simulink model "mbf_3beams"  (R2013a)
settings: Solver=VariableStepDiscrete  StartTime=0.0  StopTime=1000  RelTol=1e-3
undefined symbols (not defined in the file): pfb_coeff_gen_calc
libraries: xbsIndex_r4 x21411, casper_library_misc x306, casper_library_delays x93, casper_library_multipliers x64, casper_library_pfbs x16, simulink x16, xbsReplacements_r4 x8, xps_library x1
(part 3 of 254)

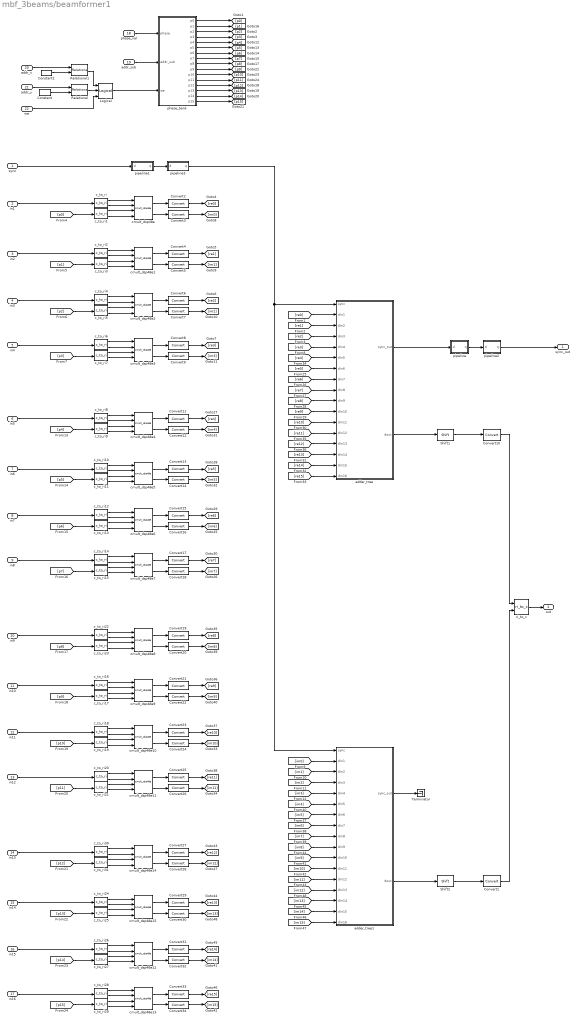
== system mbf_3beams/beamformer1 ==
  sync : Inport
  in1 : Inport  Port=2
  in2 : Inport  Port=3
  in3 : Inport  Port=4
  in4 : Inport  Port=5
  in5 : Inport  Port=6
  in6 : Inport  Port=7
  in7 : Inport  Port=8
  in8 : Inport  Port=9
  in9 : Inport  Port=10
  in10 : Inport  Port=11
  in11 : Inport  Port=12
  in12 : Inport  Port=13
  in13 : Inport  Port=14
  in14 : Inport  Port=15
  in15 : Inport  Port=16
  in16 : Inport  Port=17
  phase_val : Inport  Port=18
  addr_sub : Inport  Port=19
  addr_x : Inport  Port=20
  addr_y : Inport  Port=21
  we : Inport  Port=22
  Constant : xbsIndex_r4/Constant  const=addr_y  gui_display_data_type=Fixed-point  arith_type=Unsigned  n_bits=32  bin_pt=0  preci_type=Single  exp_width=8  frac_width=24  explicit_period=on  period=1  equ=P=C  opselect=C  inp2=PCIN>>17  opr=+  inp1=P  carry=CIN  dbl_ovrd=off  block_type=constant
  Constant1 : xbsIndex_r4/Constant  const=addr_x  gui_display_data_type=Fixed-point  arith_type=Unsigned  n_bits=32  bin_pt=0  preci_type=Single  exp_width=8  frac_width=24  explicit_period=on  period=1  equ=P=C  opselect=C  inp2=PCIN>>17  opr=+  inp1=P  carry=CIN  dbl_ovrd=off  block_type=constant
  Convert{1..34} (34 alike) : xbsIndex_r4/Convert  gui_display_data_type=Fixed-point  arith_type="Signed  (2's comp)"  n_bits=18  bin_pt=17  float_type=Single  exp_bits=8  fraction_bits=24  quantization=Truncate  overflow=Wrap  en=off  latency=2  dbl_ovrd=off  pipeline=off  block_type=convert
      settings: block_version=11.4
  Logical : xbsIndex_r4/Logical  logical_function=AND  inputs=3  en=off  latency=0  precision=Full  arith_type=Unsigned  n_bits=16  bin_pt=0  align_bp=on  dbl_ovrd=off  block_type=logical
  Relational : xbsIndex_r4/Relational  mode=a=b  en=off  latency=0  dbl_ovrd=off  block_type=relational
  Relational1 : xbsIndex_r4/Relational  mode=a=b  en=off  latency=0  dbl_ovrd=off  block_type=relational
  Shift1 : xbsIndex_r4/Shift  shift_dir=Right  shift_bits=4  en=off  latency=1  precision=Full  arith_type="Signed  (2's comp)"  n_bits=16  bin_pt=14  quantization=Truncate  overflow=Wrap  dbl_ovrd=off  block_type=shift
  Shift2 : xbsIndex_r4/Shift  shift_dir=Right  shift_bits=4  en=off  latency=1  precision=Full  arith_type="Signed  (2's comp)"  n_bits=16  bin_pt=14  quantization=Truncate  overflow=Wrap  dbl_ovrd=off  block_type=shift
  Terminator : Terminator
  adder_tree : Subsystem  AncestorBlock=casper_library_misc/adder_tree  mask(adder_tree){n_inputs=16, latency=1, adder_imp=Fabric, first_stage_hdl=on}
      mask prompts: n_inputs "Number of Inputs"; latency "Add Latency"; adder_imp "Adder implementation"; first_stage_hdl "Use behavioural HDL for first stage of adders"
      mask help:
      | Sums all inputs using a tree of adds and delays.
      |
      | Number of inputs: The number of inputs to the block to be summed
      | Add latency: The number of clock cycles to perform one addition (affects timing)..
      | Adder implementation: choice of fabric or DSP48E core, or behavioral HDL
      | Use behavioural HDL for first stage of adders: Causes the first stage of adders to be absorbed into DSP blocks if multipliers precede them (Virtex 5). Overrides Adder implementation
      mask init:
      | adder_tree_init(gcb, ...
      |     'n_inputs', n_inputs, ...
      |     'latency', latency, ...
      |     'adder_imp', adder_imp, ...
      |     'first_stage_hdl', first_stage_hdl);
      settings: AttributesFormatString=latency 4
      mask Help: eval('xlWeb(''http://casper.berkeley.edu/wiki/Adder_tree'')')
      mask TypeOptions adder_imp: Behavioral | Fabric | DSP48
  adder_tree1 : Subsystem  AncestorBlock=casper_library_misc/adder_tree  mask(adder_tree){n_inputs=16, latency=1, adder_imp=Fabric, first_stage_hdl=on}
      mask prompts: n_inputs "Number of Inputs"; latency "Add Latency"; adder_imp "Adder implementation"; first_stage_hdl "Use behavioural HDL for first stage of adders"
      mask help:
      | Sums all inputs using a tree of adds and delays.
      |
      | Number of inputs: The number of inputs to the block to be summed
      | Add latency: The number of clock cycles to perform one addition (affects timing)..
      | Adder implementation: choice of fabric or DSP48E core, or behavioral HDL
      | Use behavioural HDL for first stage of adders: Causes the first stage of adders to be absorbed into DSP blocks if multipliers precede them (Virtex 5). Overrides Adder implementation
      mask init:
      | adder_tree_init(gcb, ...
      |     'n_inputs', n_inputs, ...
      |     'latency', latency, ...
      |     'adder_imp', adder_imp, ...
      |     'first_stage_hdl', first_stage_hdl);
      settings: AttributesFormatString=latency 4
      mask Help: eval('xlWeb(''http://casper.berkeley.edu/wiki/Adder_tree'')')
      mask TypeOptions adder_imp: Behavioral | Fabric | DSP48
  c_to_ri{,1..31} (32 alike) : casper_library_misc/c_to_ri  n_bits=18  bin_pt=17
  cmult_dsp48e{,1..15} (16 alike) : casper_library_multipliers/cmult_dsp48e  n_bits_a=18  bin_pt_a=17  n_bits_b=18  bin_pt_b=17  conjugated=off  full_precision=on  n_bits_c=37  bin_pt_c=34  quantization=Truncate  overflow=Wrap  cast_latency=0
      settings: AttributesFormatString=|
      | 18_17 * 18_17 ==> 37_34
      | Latency=4
  phase_bank : Subsystem
  pipeline : Subsystem  AncestorBlock=casper_library_delays/pipeline  mask(pipeline){latency=1}
      mask help:
      | An explicitly laid-out delay line to avoid mapping to SRL16s
      | for use in pipelining to help achieve timing closure.
      mask init:
      | pipeline_init(gcb,'latency',latency);
      settings: AttributesFormatString=Latency = %<latency>
      mask Display:
      | port_label('input', 1, 'd');
      | port_label('output', 1, 'q');
      | disp(['Z^-', num2str(latency)]);
  pipeline1 : Subsystem  AncestorBlock=casper_library_delays/pipeline  mask(pipeline){latency=4}
      mask help:
      | An explicitly laid-out delay line to avoid mapping to SRL16s
      | for use in pipelining to help achieve timing closure.
      mask init:
      | pipeline_init(gcb,'latency',latency);
      settings: AttributesFormatString=Latency = %<latency>
      mask Display:
      | port_label('input', 1, 'd');
      | port_label('output', 1, 'q');
      | disp(['Z^-', num2str(latency)]);
  pipeline2 : Subsystem  AncestorBlock=casper_library_delays/pipeline  mask(pipeline){latency=2}
      mask help:
      | An explicitly laid-out delay line to avoid mapping to SRL16s
      | for use in pipelining to help achieve timing closure.
      mask init:
      | pipeline_init(gcb,'latency',latency);
      settings: AttributesFormatString=Latency = %<latency>
      mask Display:
      | port_label('input', 1, 'd');
      | port_label('output', 1, 'q');
      | disp(['Z^-', num2str(latency)]);
  pipeline3 : Subsystem  AncestorBlock=casper_library_delays/pipeline  mask(pipeline){latency=2}
      mask help:
      | An explicitly laid-out delay line to avoid mapping to SRL16s
      | for use in pipelining to help achieve timing closure.
      mask init:
      | pipeline_init(gcb,'latency',latency);
      settings: AttributesFormatString=Latency = %<latency>
      mask Display:
      | port_label('input', 1, 'd');
      | port_label('output', 1, 'q');
      | disp(['Z^-', num2str(latency)]);
  ri_to_c : casper_library_misc/ri_to_c
  sync_out : Outport
  out : Outport  Port=2
  wires:
  adder_tree1/1(sync_out) -> Terminator/1
  Constant1/1 -> Relational1/2
  Relational1/1 -> Logical/1
  addr_x/1 -> Relational1/1
  Constant/1 -> Relational/2
  Relational/1 -> Logical/2
  addr_y/1 -> Relational/1
  we/1 -> Logical/3
  [im13] -> adder_tree1/15(din14)
  [im14] -> adder_tree1/16(din15)
  [im15] -> adder_tree1/17(din16)
  [im12] -> adder_tree1/14(din13)
  [im10] -> adder_tree1/12(din11)
  [im8] -> adder_tree1/10(din9)
  [im9] -> adder_tree1/11(din10)
  [im11] -> adder_tree1/13(din12)
  [im7] -> adder_tree1/9(din8)
  [im4] -> adder_tree1/6(din5)
  [im5] -> adder_tree1/7(din6)
  [im6] -> adder_tree1/8(din7)
  [im3] -> adder_tree1/5(din4)
  [im2] -> adder_tree1/4(din3)
  [im1] -> adder_tree1/3(din2)
  [im0] -> adder_tree1/2(din1)
  pipeline3/1(q) -> adder_tree/1(sync), adder_tree1/1(sync)
  [re14] -> adder_tree/16(din15)
  [re13] -> adder_tree/15(din14)
  [re8] -> adder_tree/10(din9)
  [re5] -> adder_tree/7(din6)
  [re11] -> adder_tree/13(din12)
  [re10] -> adder_tree/12(din11)
  [re6] -> adder_tree/8(din7)
  [re4] -> adder_tree/6(din5)
  [re7] -> adder_tree/9(din8)
  [re9] -> adder_tree/11(din10)
  [re12] -> adder_tree/14(din13)
  [re15] -> adder_tree/17(din16)
  c_to_ri24/2 -> cmult_dsp48e15/2
  in14/1 -> c_to_ri24/1
  cmult_dsp48e15/1 -> Convert29/1
  Convert29/1 -> [re13]
  cmult_dsp48e15/2 -> Convert30/1
  c_to_ri24/1 -> cmult_dsp48e15/1
  c_to_ri25/2 -> cmult_dsp48e15/4
  Convert30/1 -> [im13]
  c_to_ri31/1 -> cmult_dsp48e14/3
  c_to_ri30/2 -> cmult_dsp48e14/2
  in13/1 -> c_to_ri30/1
  [p12] -> c_to_ri31/1
  cmult_dsp48e14/1 -> Convert27/1
  Convert27/1 -> [re12]
  cmult_dsp48e14/2 -> Convert28/1
  c_to_ri30/1 -> cmult_dsp48e14/1
  c_to_ri31/2 -> cmult_dsp48e14/4
  Convert28/1 -> [im12]
  c_to_ri29/1 -> cmult_dsp48e13/3
  c_to_ri28/2 -> cmult_dsp48e13/2
  in16/1 -> c_to_ri28/1
  [p15] -> c_to_ri29/1
  cmult_dsp48e13/1 -> Convert33/1
  Convert33/1 -> [re15]
  cmult_dsp48e13/2 -> Convert34/1
  c_to_ri28/1 -> cmult_dsp48e13/1
  c_to_ri29/2 -> cmult_dsp48e13/4
  Convert34/1 -> [im15]
  c_to_ri27/1 -> cmult_dsp48e12/3
  c_to_ri26/2 -> cmult_dsp48e12/2
  in15/1 -> c_to_ri26/1
  [p14] -> c_to_ri27/1
  cmult_dsp48e12/1 -> Convert31/1
  Convert31/1 -> [re14]
  cmult_dsp48e12/2 -> Convert32/1
  c_to_ri26/1 -> cmult_dsp48e12/1
  c_to_ri27/2 -> cmult_dsp48e12/4
  Convert32/1 -> [im14]
  c_to_ri25/1 -> cmult_dsp48e15/3
  [p13] -> c_to_ri25/1
  c_to_ri16/2 -> cmult_dsp48e9/2
  in10/1 -> c_to_ri16/1
  cmult_dsp48e9/1 -> Convert21/1
  Convert21/1 -> [re9]
  cmult_dsp48e9/2 -> Convert22/1
  c_to_ri16/1 -> cmult_dsp48e9/1
  c_to_ri17/2 -> cmult_dsp48e9/4
  Convert22/1 -> [im9]
  c_to_ri23/1 -> cmult_dsp48e8/3
  c_to_ri22/2 -> cmult_dsp48e8/2
  in9/1 -> c_to_ri22/1
  [p8] -> c_to_ri23/1
  cmult_dsp48e8/1 -> Convert19/1
  Convert19/1 -> [re8]
  cmult_dsp48e8/2 -> Convert20/1
  c_to_ri22/1 -> cmult_dsp48e8/1
  c_to_ri23/2 -> cmult_dsp48e8/4
  Convert20/1 -> [im8]
  c_to_ri21/1 -> cmult_dsp48e11/3
  c_to_ri20/2 -> cmult_dsp48e11/2
  in12/1 -> c_to_ri20/1
  [p11] -> c_to_ri21/1
  cmult_dsp48e11/1 -> Convert25/1
  Convert25/1 -> [re11]
  cmult_dsp48e11/2 -> Convert26/1
  c_to_ri20/1 -> cmult_dsp48e11/1
  c_to_ri21/2 -> cmult_dsp48e11/4
  Convert26/1 -> [im11]
  c_to_ri19/1 -> cmult_dsp48e10/3
  c_to_ri18/2 -> cmult_dsp48e10/2
  in11/1 -> c_to_ri18/1
  [p10] -> c_to_ri19/1
  cmult_dsp48e10/1 -> Convert23/1
  Convert23/1 -> [re10]
  cmult_dsp48e10/2 -> Convert24/1
  c_to_ri18/1 -> cmult_dsp48e10/1
  c_to_ri19/2 -> cmult_dsp48e10/4
  Convert24/1 -> [im10]
  c_to_ri17/1 -> cmult_dsp48e9/3
  [p9] -> c_to_ri17/1
  c_to_ri10/2 -> cmult_dsp48e5/2
  in6/1 -> c_to_ri10/1
  cmult_dsp48e5/1 -> Convert13/1
  Convert13/1 -> [re5]
  cmult_dsp48e5/2 -> Convert14/1
  c_to_ri10/1 -> cmult_dsp48e5/1
  c_to_ri11/2 -> cmult_dsp48e5/4
  Convert14/1 -> [im5]
  c_to_ri9/1 -> cmult_dsp48e4/3
  c_to_ri8/2 -> cmult_dsp48e4/2
  in5/1 -> c_to_ri8/1
  [p4] -> c_to_ri9/1
  cmult_dsp48e4/1 -> Convert11/1
  Convert11/1 -> [re4]
  cmult_dsp48e4/2 -> Convert12/1
  c_to_ri8/1 -> cmult_dsp48e4/1
  c_to_ri9/2 -> cmult_dsp48e4/4
  Convert12/1 -> [im4]
  c_to_ri15/1 -> cmult_dsp48e7/3
  c_to_ri14/2 -> cmult_dsp48e7/2
  in8/1 -> c_to_ri14/1
  [p7] -> c_to_ri15/1
  cmult_dsp48e7/1 -> Convert17/1
  Convert17/1 -> [re7]
  cmult_dsp48e7/2 -> Convert18/1
  c_to_ri14/1 -> cmult_dsp48e7/1
  c_to_ri15/2 -> cmult_dsp48e7/4
  Convert18/1 -> [im7]
  c_to_ri13/1 -> cmult_dsp48e6/3
  c_to_ri12/2 -> cmult_dsp48e6/2
  in7/1 -> c_to_ri12/1
  [p6] -> c_to_ri13/1
  cmult_dsp48e6/1 -> Convert15/1
  Convert15/1 -> [re6]
  cmult_dsp48e6/2 -> Convert16/1
  c_to_ri12/1 -> cmult_dsp48e6/1
  c_to_ri13/2 -> cmult_dsp48e6/4
  Convert16/1 -> [im6]
  c_to_ri11/1 -> cmult_dsp48e5/3
  [p5] -> c_to_ri11/1
  Logical/1 -> phase_bank/3(we)
  addr_sub/1 -> phase_bank/2(addr_sub)
  phase_val/1 -> phase_bank/1(phase)
  ri_to_c/1 -> out/1
  pipeline2/1(q) -> sync_out/1
  Convert10/1 -> ri_to_c/1
  Convert1/1 -> ri_to_c/2
  Shift2/1 -> Convert1/1
  Shift1/1 -> Convert10/1
  pipeline/1(q) -> pipeline2/1(d)
  [re0] -> adder_tree/2(din1)
  [re1] -> adder_tree/3(din2)
  [re2] -> adder_tree/4(din3)
  [re3] -> adder_tree/5(din4)
  adder_tree/1(sync_out) -> pipeline/1(d)
  adder_tree1/2(dout) -> Shift2/1
  adder_tree/2(dout) -> Shift1/1
  c_to_ri2/2 -> cmult_dsp48e1/2
  in2/1 -> c_to_ri2/1
  cmult_dsp48e1/1 -> Convert4/1
  Convert4/1 -> [re1]
  cmult_dsp48e1/2 -> Convert5/1
  c_to_ri2/1 -> cmult_dsp48e1/1
  c_to_ri3/2 -> cmult_dsp48e1/4
  Convert5/1 -> [im1]
  c_to_ri1/1 -> cmult_dsp48e/3
  c_to_ri/2 -> cmult_dsp48e/2
  in1/1 -> c_to_ri/1
  [p0] -> c_to_ri1/1
  cmult_dsp48e/1 -> Convert2/1
  Convert2/1 -> [re0]
  cmult_dsp48e/2 -> Convert3/1
  c_to_ri/1 -> cmult_dsp48e/1
  c_to_ri1/2 -> cmult_dsp48e/4
  Convert3/1 -> [im0]
  pipeline1/1(q) -> pipeline3/1(d)
  sync/1 -> pipeline1/1(d)
  c_to_ri7/1 -> cmult_dsp48e3/3
  c_to_ri6/2 -> cmult_dsp48e3/2
  in4/1 -> c_to_ri6/1
  [p3] -> c_to_ri7/1
  cmult_dsp48e3/1 -> Convert8/1
  Convert8/1 -> [re3]
  cmult_dsp48e3/2 -> Convert9/1
  c_to_ri6/1 -> cmult_dsp48e3/1
  c_to_ri7/2 -> cmult_dsp48e3/4
  Convert9/1 -> [im3]
  c_to_ri5/1 -> cmult_dsp48e2/3
  c_to_ri4/2 -> cmult_dsp48e2/2
  in3/1 -> c_to_ri4/1
  [p2] -> c_to_ri5/1
  cmult_dsp48e2/1 -> Convert6/1
  Convert6/1 -> [re2]
  cmult_dsp48e2/2 -> Convert7/1
  c_to_ri4/1 -> cmult_dsp48e2/1
  c_to_ri5/2 -> cmult_dsp48e2/4
  Convert7/1 -> [im2]
  c_to_ri3/1 -> cmult_dsp48e1/3
  [p1] -> c_to_ri3/1
  phase_bank/1(p0) -> [p0]
  phase_bank/2(p1) -> [p1]
  phase_bank/3(p2) -> [p2]
  phase_bank/4(p3) -> [p3]
  phase_bank/5(p4) -> [p4]
  phase_bank/6(p5) -> [p5]
  phase_bank/7(p6) -> [p6]
  phase_bank/8(p7) -> [p7]
  phase_bank/9(p8) -> [p8]
  phase_bank/10(p9) -> [p9]
  phase_bank/11(p10) -> [p10]
  phase_bank/12(p11) -> [p11]
  phase_bank/13(p12) -> [p12]
  phase_bank/14(p13) -> [p13]
  phase_bank/15(p14) -> [p14]
  phase_bank/16(p15) -> [p15]

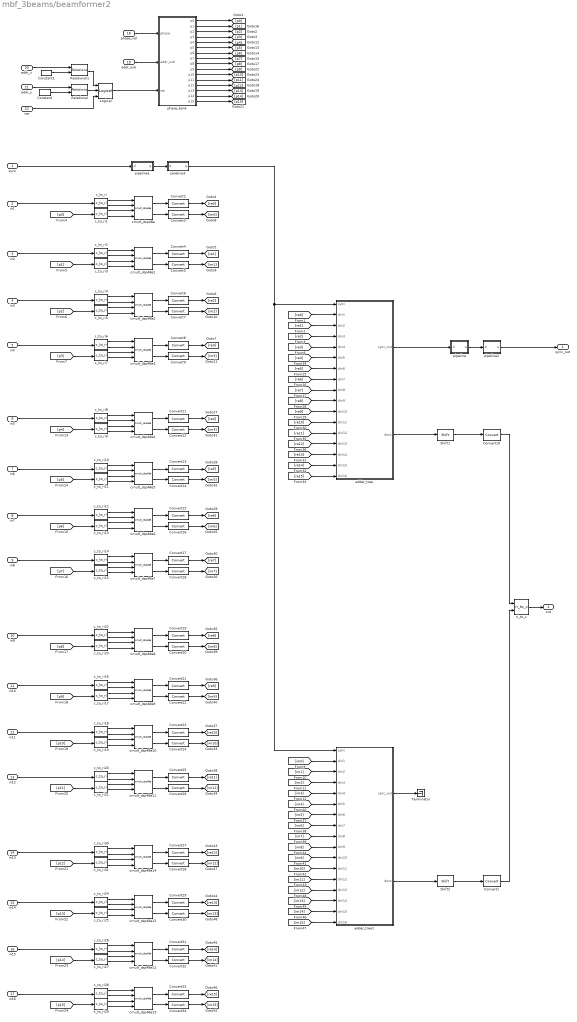
== system mbf_3beams/beamformer2 ==
  sync : Inport
  in1 : Inport  Port=2
  in2 : Inport  Port=3
  in3 : Inport  Port=4
  in4 : Inport  Port=5
  in5 : Inport  Port=6
  in6 : Inport  Port=7
  in7 : Inport  Port=8
  in8 : Inport  Port=9
  in9 : Inport  Port=10
  in10 : Inport  Port=11
  in11 : Inport  Port=12
  in12 : Inport  Port=13
  in13 : Inport  Port=14
  in14 : Inport  Port=15
  in15 : Inport  Port=16
  in16 : Inport  Port=17
  phase_val : Inport  Port=18
  addr_sub : Inport  Port=19
  addr_x : Inport  Port=20
  addr_y : Inport  Port=21
  we : Inport  Port=22
  Constant : xbsIndex_r4/Constant  const=addr_y  gui_display_data_type=Fixed-point  arith_type=Unsigned  n_bits=32  bin_pt=0  preci_type=Single  exp_width=8  frac_width=24  explicit_period=on  period=1  equ=P=C  opselect=C  inp2=PCIN>>17  opr=+  inp1=P  carry=CIN  dbl_ovrd=off  block_type=constant
  Constant1 : xbsIndex_r4/Constant  const=addr_x  gui_display_data_type=Fixed-point  arith_type=Unsigned  n_bits=32  bin_pt=0  preci_type=Single  exp_width=8  frac_width=24  explicit_period=on  period=1  equ=P=C  opselect=C  inp2=PCIN>>17  opr=+  inp1=P  carry=CIN  dbl_ovrd=off  block_type=constant
  Convert{1..34} (34 alike) : xbsIndex_r4/Convert  gui_display_data_type=Fixed-point  arith_type="Signed  (2's comp)"  n_bits=18  bin_pt=17  float_type=Single  exp_bits=8  fraction_bits=24  quantization=Truncate  overflow=Wrap  en=off  latency=2  dbl_ovrd=off  pipeline=off  block_type=convert
      settings: block_version=11.4
  Logical : xbsIndex_r4/Logical  logical_function=AND  inputs=3  en=off  latency=0  precision=Full  arith_type=Unsigned  n_bits=16  bin_pt=0  align_bp=on  dbl_ovrd=off  block_type=logical
  Relational : xbsIndex_r4/Relational  mode=a=b  en=off  latency=0  dbl_ovrd=off  block_type=relational
  Relational1 : xbsIndex_r4/Relational  mode=a=b  en=off  latency=0  dbl_ovrd=off  block_type=relational
  Shift1 : xbsIndex_r4/Shift  shift_dir=Right  shift_bits=4  en=off  latency=1  precision=Full  arith_type="Signed  (2's comp)"  n_bits=16  bin_pt=14  quantization=Truncate  overflow=Wrap  dbl_ovrd=off  block_type=shift
  Shift2 : xbsIndex_r4/Shift  shift_dir=Right  shift_bits=4  en=off  latency=1  precision=Full  arith_type="Signed  (2's comp)"  n_bits=16  bin_pt=14  quantization=Truncate  overflow=Wrap  dbl_ovrd=off  block_type=shift
  Terminator : Terminator
  adder_tree : Subsystem  AncestorBlock=casper_library_misc/adder_tree  mask(adder_tree){n_inputs=16, latency=1, adder_imp=Fabric, first_stage_hdl=on}
      mask prompts: n_inputs "Number of Inputs"; latency "Add Latency"; adder_imp "Adder implementation"; first_stage_hdl "Use behavioural HDL for first stage of adders"
      mask help:
      | Sums all inputs using a tree of adds and delays.
      |
      | Number of inputs: The number of inputs to the block to be summed
      | Add latency: The number of clock cycles to perform one addition (affects timing)..
      | Adder implementation: choice of fabric or DSP48E core, or behavioral HDL
      | Use behavioural HDL for first stage of adders: Causes the first stage of adders to be absorbed into DSP blocks if multipliers precede them (Virtex 5). Overrides Adder implementation
      mask init:
      | adder_tree_init(gcb, ...
      |     'n_inputs', n_inputs, ...
      |     'latency', latency, ...
      |     'adder_imp', adder_imp, ...
      |     'first_stage_hdl', first_stage_hdl);
      settings: AttributesFormatString=latency 4
      mask Help: eval('xlWeb(''http://casper.berkeley.edu/wiki/Adder_tree'')')
      mask TypeOptions adder_imp: Behavioral | Fabric | DSP48
  adder_tree1 : Subsystem  AncestorBlock=casper_library_misc/adder_tree  mask(adder_tree){n_inputs=16, latency=1, adder_imp=Fabric, first_stage_hdl=on}
      mask prompts: n_inputs "Number of Inputs"; latency "Add Latency"; adder_imp "Adder implementation"; first_stage_hdl "Use behavioural HDL for first stage of adders"
      mask help:
      | Sums all inputs using a tree of adds and delays.
      |
      | Number of inputs: The number of inputs to the block to be summed
      | Add latency: The number of clock cycles to perform one addition (affects timing)..
      | Adder implementation: choice of fabric or DSP48E core, or behavioral HDL
      | Use behavioural HDL for first stage of adders: Causes the first stage of adders to be absorbed into DSP blocks if multipliers precede them (Virtex 5). Overrides Adder implementation
      mask init:
      | adder_tree_init(gcb, ...
      |     'n_inputs', n_inputs, ...
      |     'latency', latency, ...
      |     'adder_imp', adder_imp, ...
      |     'first_stage_hdl', first_stage_hdl);
      settings: AttributesFormatString=latency 4
      mask Help: eval('xlWeb(''http://casper.berkeley.edu/wiki/Adder_tree'')')
      mask TypeOptions adder_imp: Behavioral | Fabric | DSP48
  c_to_ri{,1..31} (32 alike) : casper_library_misc/c_to_ri  n_bits=18  bin_pt=17
  cmult_dsp48e{,1..15} (16 alike) : casper_library_multipliers/cmult_dsp48e  n_bits_a=18  bin_pt_a=17  n_bits_b=18  bin_pt_b=17  conjugated=off  full_precision=on  n_bits_c=37  bin_pt_c=34  quantization=Truncate  overflow=Wrap  cast_latency=0
      settings: AttributesFormatString=|
      | 18_17 * 18_17 ==> 37_34
      | Latency=4
  phase_bank : Subsystem
  pipeline : Subsystem  AncestorBlock=casper_library_delays/pipeline  mask(pipeline){latency=1}
      mask help:
      | An explicitly laid-out delay line to avoid mapping to SRL16s
      | for use in pipelining to help achieve timing closure.
      mask init:
      | pipeline_init(gcb,'latency',latency);
      settings: AttributesFormatString=Latency = %<latency>
      mask Display:
      | port_label('input', 1, 'd');
      | port_label('output', 1, 'q');
      | disp(['Z^-', num2str(latency)]);
  pipeline1 : Subsystem  AncestorBlock=casper_library_delays/pipeline  mask(pipeline){latency=4}
      mask help:
      | An explicitly laid-out delay line to avoid mapping to SRL16s
      | for use in pipelining to help achieve timing closure.
      mask init:
      | pipeline_init(gcb,'latency',latency);
      settings: AttributesFormatString=Latency = %<latency>
      mask Display:
      | port_label('input', 1, 'd');
      | port_label('output', 1, 'q');
      | disp(['Z^-', num2str(latency)]);
  pipeline2 : Subsystem  AncestorBlock=casper_library_delays/pipeline  mask(pipeline){latency=2}
      mask help:
      | An explicitly laid-out delay line to avoid mapping to SRL16s
      | for use in pipelining to help achieve timing closure.
      mask init:
      | pipeline_init(gcb,'latency',latency);
      settings: AttributesFormatString=Latency = %<latency>
      mask Display:
      | port_label('input', 1, 'd');
      | port_label('output', 1, 'q');
      | disp(['Z^-', num2str(latency)]);
  pipeline3 : Subsystem  AncestorBlock=casper_library_delays/pipeline  mask(pipeline){latency=2}
      mask help:
      | An explicitly laid-out delay line to avoid mapping to SRL16s
      | for use in pipelining to help achieve timing closure.
      mask init:
      | pipeline_init(gcb,'latency',latency);
      settings: AttributesFormatString=Latency = %<latency>
      mask Display:
      | port_label('input', 1, 'd');
      | port_label('output', 1, 'q');
      | disp(['Z^-', num2str(latency)]);
  ri_to_c : casper_library_misc/ri_to_c
  sync_out : Outport
  out : Outport  Port=2
  wires:
  phase_bank/16(p15) -> [p15]
  phase_bank/15(p14) -> [p14]
  phase_bank/14(p13) -> [p13]
  phase_bank/13(p12) -> [p12]
  phase_bank/12(p11) -> [p11]
  phase_bank/11(p10) -> [p10]
  phase_bank/10(p9) -> [p9]
  phase_bank/9(p8) -> [p8]
  phase_bank/8(p7) -> [p7]
  phase_bank/7(p6) -> [p6]
  phase_bank/6(p5) -> [p5]
  phase_bank/5(p4) -> [p4]
  phase_bank/4(p3) -> [p3]
  phase_bank/3(p2) -> [p2]
  phase_bank/2(p1) -> [p1]
  phase_bank/1(p0) -> [p0]
  [p1] -> c_to_ri3/1
  c_to_ri3/1 -> cmult_dsp48e1/3
  Convert7/1 -> [im2]
  c_to_ri5/2 -> cmult_dsp48e2/4
  c_to_ri4/1 -> cmult_dsp48e2/1
  cmult_dsp48e2/2 -> Convert7/1
  Convert6/1 -> [re2]
  cmult_dsp48e2/1 -> Convert6/1
  [p2] -> c_to_ri5/1
  in3/1 -> c_to_ri4/1
  c_to_ri4/2 -> cmult_dsp48e2/2
  c_to_ri5/1 -> cmult_dsp48e2/3
  Convert9/1 -> [im3]
  c_to_ri7/2 -> cmult_dsp48e3/4
  c_to_ri6/1 -> cmult_dsp48e3/1
  cmult_dsp48e3/2 -> Convert9/1
  Convert8/1 -> [re3]
  cmult_dsp48e3/1 -> Convert8/1
  [p3] -> c_to_ri7/1
  in4/1 -> c_to_ri6/1
  c_to_ri6/2 -> cmult_dsp48e3/2
  c_to_ri7/1 -> cmult_dsp48e3/3
  sync/1 -> pipeline1/1(d)
  pipeline1/1(q) -> pipeline3/1(d)
  Convert3/1 -> [im0]
  c_to_ri1/2 -> cmult_dsp48e/4
  c_to_ri/1 -> cmult_dsp48e/1
  cmult_dsp48e/2 -> Convert3/1
  Convert2/1 -> [re0]
  cmult_dsp48e/1 -> Convert2/1
  [p0] -> c_to_ri1/1
  in1/1 -> c_to_ri/1
  c_to_ri/2 -> cmult_dsp48e/2
  c_to_ri1/1 -> cmult_dsp48e/3
  Convert5/1 -> [im1]
  c_to_ri3/2 -> cmult_dsp48e1/4
  c_to_ri2/1 -> cmult_dsp48e1/1
  cmult_dsp48e1/2 -> Convert5/1
  Convert4/1 -> [re1]
  cmult_dsp48e1/1 -> Convert4/1
  in2/1 -> c_to_ri2/1
  c_to_ri2/2 -> cmult_dsp48e1/2
  adder_tree/2(dout) -> Shift1/1
  adder_tree1/2(dout) -> Shift2/1
  adder_tree/1(sync_out) -> pipeline/1(d)
  [re3] -> adder_tree/5(din4)
  [re2] -> adder_tree/4(din3)
  [re1] -> adder_tree/3(din2)
  [re0] -> adder_tree/2(din1)
  pipeline/1(q) -> pipeline2/1(d)
  Shift1/1 -> Convert10/1
  Shift2/1 -> Convert1/1
  Convert1/1 -> ri_to_c/2
  Convert10/1 -> ri_to_c/1
  pipeline2/1(q) -> sync_out/1
  ri_to_c/1 -> out/1
  phase_val/1 -> phase_bank/1(phase)
  addr_sub/1 -> phase_bank/2(addr_sub)
  Logical/1 -> phase_bank/3(we)
  [p5] -> c_to_ri11/1
  c_to_ri11/1 -> cmult_dsp48e5/3
  Convert16/1 -> [im6]
  c_to_ri13/2 -> cmult_dsp48e6/4
  c_to_ri12/1 -> cmult_dsp48e6/1
  cmult_dsp48e6/2 -> Convert16/1
  Convert15/1 -> [re6]
  cmult_dsp48e6/1 -> Convert15/1
  [p6] -> c_to_ri13/1
  in7/1 -> c_to_ri12/1
  c_to_ri12/2 -> cmult_dsp48e6/2
  c_to_ri13/1 -> cmult_dsp48e6/3
  Convert18/1 -> [im7]
  c_to_ri15/2 -> cmult_dsp48e7/4
  c_to_ri14/1 -> cmult_dsp48e7/1
  cmult_dsp48e7/2 -> Convert18/1
  Convert17/1 -> [re7]
  cmult_dsp48e7/1 -> Convert17/1
  [p7] -> c_to_ri15/1
  in8/1 -> c_to_ri14/1
  c_to_ri14/2 -> cmult_dsp48e7/2
  c_to_ri15/1 -> cmult_dsp48e7/3
  Convert12/1 -> [im4]
  c_to_ri9/2 -> cmult_dsp48e4/4
  c_to_ri8/1 -> cmult_dsp48e4/1
  cmult_dsp48e4/2 -> Convert12/1
  Convert11/1 -> [re4]
  cmult_dsp48e4/1 -> Convert11/1
  [p4] -> c_to_ri9/1
  in5/1 -> c_to_ri8/1
  c_to_ri8/2 -> cmult_dsp48e4/2
  c_to_ri9/1 -> cmult_dsp48e4/3
  Convert14/1 -> [im5]
  c_to_ri11/2 -> cmult_dsp48e5/4
  c_to_ri10/1 -> cmult_dsp48e5/1
  cmult_dsp48e5/2 -> Convert14/1
  Convert13/1 -> [re5]
  cmult_dsp48e5/1 -> Convert13/1
  in6/1 -> c_to_ri10/1
  c_to_ri10/2 -> cmult_dsp48e5/2
  [p9] -> c_to_ri17/1
  c_to_ri17/1 -> cmult_dsp48e9/3
  Convert24/1 -> [im10]
  c_to_ri19/2 -> cmult_dsp48e10/4
  c_to_ri18/1 -> cmult_dsp48e10/1
  cmult_dsp48e10/2 -> Convert24/1
  Convert23/1 -> [re10]
  cmult_dsp48e10/1 -> Convert23/1
  [p10] -> c_to_ri19/1
  in11/1 -> c_to_ri18/1
  c_to_ri18/2 -> cmult_dsp48e10/2
  c_to_ri19/1 -> cmult_dsp48e10/3
  Convert26/1 -> [im11]
  c_to_ri21/2 -> cmult_dsp48e11/4
  c_to_ri20/1 -> cmult_dsp48e11/1
  cmult_dsp48e11/2 -> Convert26/1
  Convert25/1 -> [re11]
  cmult_dsp48e11/1 -> Convert25/1
  [p11] -> c_to_ri21/1
  in12/1 -> c_to_ri20/1
  c_to_ri20/2 -> cmult_dsp48e11/2
  c_to_ri21/1 -> cmult_dsp48e11/3
  Convert20/1 -> [im8]
  c_to_ri23/2 -> cmult_dsp48e8/4
  c_to_ri22/1 -> cmult_dsp48e8/1
  cmult_dsp48e8/2 -> Convert20/1
  Convert19/1 -> [re8]
  cmult_dsp48e8/1 -> Convert19/1
  [p8] -> c_to_ri23/1
  in9/1 -> c_to_ri22/1
  c_to_ri22/2 -> cmult_dsp48e8/2
  c_to_ri23/1 -> cmult_dsp48e8/3
  Convert22/1 -> [im9]
  c_to_ri17/2 -> cmult_dsp48e9/4
  c_to_ri16/1 -> cmult_dsp48e9/1
  cmult_dsp48e9/2 -> Convert22/1
  Convert21/1 -> [re9]
  cmult_dsp48e9/1 -> Convert21/1
  in10/1 -> c_to_ri16/1
  c_to_ri16/2 -> cmult_dsp48e9/2
  [p13] -> c_to_ri25/1
  c_to_ri25/1 -> cmult_dsp48e15/3
  Convert32/1 -> [im14]
  c_to_ri27/2 -> cmult_dsp48e12/4
  c_to_ri26/1 -> cmult_dsp48e12/1
  cmult_dsp48e12/2 -> Convert32/1
  Convert31/1 -> [re14]
  cmult_dsp48e12/1 -> Convert31/1
  [p14] -> c_to_ri27/1
  in15/1 -> c_to_ri26/1
  c_to_ri26/2 -> cmult_dsp48e12/2
  c_to_ri27/1 -> cmult_dsp48e12/3
  Convert34/1 -> [im15]
  c_to_ri29/2 -> cmult_dsp48e13/4
  c_to_ri28/1 -> cmult_dsp48e13/1
  cmult_dsp48e13/2 -> Convert34/1
  Convert33/1 -> [re15]
  cmult_dsp48e13/1 -> Convert33/1
  [p15] -> c_to_ri29/1
  in16/1 -> c_to_ri28/1
  c_to_ri28/2 -> cmult_dsp48e13/2
  c_to_ri29/1 -> cmult_dsp48e13/3
  Convert28/1 -> [im12]
  c_to_ri31/2 -> cmult_dsp48e14/4
  c_to_ri30/1 -> cmult_dsp48e14/1
  cmult_dsp48e14/2 -> Convert28/1
  Convert27/1 -> [re12]
  cmult_dsp48e14/1 -> Convert27/1
  [p12] -> c_to_ri31/1
  in13/1 -> c_to_ri30/1
  c_to_ri30/2 -> cmult_dsp48e14/2
  c_to_ri31/1 -> cmult_dsp48e14/3
  Convert30/1 -> [im13]
  c_to_ri25/2 -> cmult_dsp48e15/4
  c_to_ri24/1 -> cmult_dsp48e15/1
  cmult_dsp48e15/2 -> Convert30/1
  Convert29/1 -> [re13]
  cmult_dsp48e15/1 -> Convert29/1
  in14/1 -> c_to_ri24/1
  c_to_ri24/2 -> cmult_dsp48e15/2
  [re15] -> adder_tree/17(din16)
  [re12] -> adder_tree/14(din13)
  [re9] -> adder_tree/11(din10)
  [re7] -> adder_tree/9(din8)
  [re4] -> adder_tree/6(din5)
  [re6] -> adder_tree/8(din7)
  [re10] -> adder_tree/12(din11)
  [re11] -> adder_tree/13(din12)
  [re5] -> adder_tree/7(din6)
  [re8] -> adder_tree/10(din9)
  [re13] -> adder_tree/15(din14)
  [re14] -> adder_tree/16(din15)
  pipeline3/1(q) -> adder_tree1/1(sync), adder_tree/1(sync)
  [im0] -> adder_tree1/2(din1)
  [im1] -> adder_tree1/3(din2)
  [im2] -> adder_tree1/4(din3)
  [im3] -> adder_tree1/5(din4)
  [im6] -> adder_tree1/8(din7)
  [im5] -> adder_tree1/7(din6)
  [im4] -> adder_tree1/6(din5)
  [im7] -> adder_tree1/9(din8)
  [im11] -> adder_tree1/13(din12)
  [im9] -> adder_tree1/11(din10)
  [im8] -> adder_tree1/10(din9)
  [im10] -> adder_tree1/12(din11)
  [im12] -> adder_tree1/14(din13)
  [im15] -> adder_tree1/17(din16)
  [im14] -> adder_tree1/16(din15)
  [im13] -> adder_tree1/15(din14)
  we/1 -> Logical/3
  addr_y/1 -> Relational/1
  Relational/1 -> Logical/2
  Constant/1 -> Relational/2
  addr_x/1 -> Relational1/1
  Relational1/1 -> Logical/1
  Constant1/1 -> Relational1/2
  adder_tree1/1(sync_out) -> Terminator/1

== system mbf_3beams/bf_acc_len ==
  io_delay : xbsIndex_r4/Delay  rst=off  infoeditControl=Selection of Reset will increase slice count due to use of real FFs and instead of SRLs  en=off  latency=0  dbl_ovrd=off  reg_retiming=on  block_type=delay
  mbf_3beams_bf_acc_len_user_data_out : xbsIndex_r4/Gateway In  gui_display_data_type=Fixed-point  arith_type=Unsigned  n_bits=32  bin_pt=0  preci_type=Single  exp_width=8  frac_width=24  quantization="Round  (unbiased: +/- Inf)"  overflow=Saturate  period=1  dbl_ovrd=off  timing_constraint=None  locs_specified=off  LOCs={}  UseAsADC=off  ADCChannel='1'  inherit_from_input=off  hdl_port=on  block_type=gatewayin
  reint1 : xbsIndex_r4/Reinterpret  force_arith_type=on  arith_type=Unsigned  force_bin_pt=on  bin_pt=0  block_type=reinterpret
  sim_1 : Constant  Value=0
  slice_reg : xbsIndex_r4/Slice  nbits=32  boolean_output=off  mode=Lower Bit Location + Width  bit1=0  base1=MSB of Input  bit0=0  base0=LSB of Input  dbl_ovrd=off  block_type=slice
  in_reg : Outport
  wires:
  mbf_3beams_bf_acc_len_user_data_out/1 -> io_delay/1
  sim_1/1 -> mbf_3beams_bf_acc_len_user_data_out/1
  io_delay/1 -> slice_reg/1
  slice_reg/1 -> reint1/1
  reint1/1 -> in_reg/1

== system mbf_3beams/bf_addr_sub ==
  io_delay : xbsIndex_r4/Delay  rst=off  infoeditControl=Selection of Reset will increase slice count due to use of real FFs and instead of SRLs  en=off  latency=0  dbl_ovrd=off  reg_retiming=on  block_type=delay
  mbf_3beams_bf_addr_sub_user_data_out : xbsIndex_r4/Gateway In  gui_display_data_type=Fixed-point  arith_type=Unsigned  n_bits=32  bin_pt=0  preci_type=Single  exp_width=8  frac_width=24  quantization="Round  (unbiased: +/- Inf)"  overflow=Saturate  period=1  dbl_ovrd=off  timing_constraint=None  locs_specified=off  LOCs={}  UseAsADC=off  ADCChannel='1'  inherit_from_input=off  hdl_port=on  block_type=gatewayin
  reint1 : xbsIndex_r4/Reinterpret  force_arith_type=on  arith_type=Unsigned  force_bin_pt=on  bin_pt=0  block_type=reinterpret
  sim_1 : Constant  Value=0
  slice_reg : xbsIndex_r4/Slice  nbits=32  boolean_output=off  mode=Lower Bit Location + Width  bit1=0  base1=MSB of Input  bit0=0  base0=LSB of Input  dbl_ovrd=off  block_type=slice
  in_reg : Outport
  wires:
  mbf_3beams_bf_addr_sub_user_data_out/1 -> io_delay/1
  sim_1/1 -> mbf_3beams_bf_addr_sub_user_data_out/1
  io_delay/1 -> slice_reg/1
  slice_reg/1 -> reint1/1
  reint1/1 -> in_reg/1

== system mbf_3beams/bf_addr_x ==
  io_delay : xbsIndex_r4/Delay  rst=off  infoeditControl=Selection of Reset will increase slice count due to use of real FFs and instead of SRLs  en=off  latency=0  dbl_ovrd=off  reg_retiming=on  block_type=delay
  mbf_3beams_bf_addr_x_user_data_out : xbsIndex_r4/Gateway In  gui_display_data_type=Fixed-point  arith_type=Unsigned  n_bits=32  bin_pt=0  preci_type=Single  exp_width=8  frac_width=24  quantization="Round  (unbiased: +/- Inf)"  overflow=Saturate  period=1  dbl_ovrd=off  timing_constraint=None  locs_specified=off  LOCs={}  UseAsADC=off  ADCChannel='1'  inherit_from_input=off  hdl_port=on  block_type=gatewayin
  reint1 : xbsIndex_r4/Reinterpret  force_arith_type=on  arith_type=Unsigned  force_bin_pt=on  bin_pt=0  block_type=reinterpret
  sim_1 : Constant  Value=0
  slice_reg : xbsIndex_r4/Slice  nbits=32  boolean_output=off  mode=Lower Bit Location + Width  bit1=0  base1=MSB of Input  bit0=0  base0=LSB of Input  dbl_ovrd=off  block_type=slice
  in_reg : Outport
  wires:
  mbf_3beams_bf_addr_x_user_data_out/1 -> io_delay/1
  sim_1/1 -> mbf_3beams_bf_addr_x_user_data_out/1
  io_delay/1 -> slice_reg/1
  slice_reg/1 -> reint1/1
  reint1/1 -> in_reg/1

== system mbf_3beams/bf_addr_y ==
  io_delay : xbsIndex_r4/Delay  rst=off  infoeditControl=Selection of Reset will increase slice count due to use of real FFs and instead of SRLs  en=off  latency=0  dbl_ovrd=off  reg_retiming=on  block_type=delay
  mbf_3beams_bf_addr_y_user_data_out : xbsIndex_r4/Gateway In  gui_display_data_type=Fixed-point  arith_type=Unsigned  n_bits=32  bin_pt=0  preci_type=Single  exp_width=8  frac_width=24  quantization="Round  (unbiased: +/- Inf)"  overflow=Saturate  period=1  dbl_ovrd=off  timing_constraint=None  locs_specified=off  LOCs={}  UseAsADC=off  ADCChannel='1'  inherit_from_input=off  hdl_port=on  block_type=gatewayin
  reint1 : xbsIndex_r4/Reinterpret  force_arith_type=on  arith_type=Unsigned  force_bin_pt=on  bin_pt=0  block_type=reinterpret
  sim_1 : Constant  Value=0
  slice_reg : xbsIndex_r4/Slice  nbits=32  boolean_output=off  mode=Lower Bit Location + Width  bit1=0  base1=MSB of Input  bit0=0  base0=LSB of Input  dbl_ovrd=off  block_type=slice
  in_reg : Outport
  wires:
  mbf_3beams_bf_addr_y_user_data_out/1 -> io_delay/1
  sim_1/1 -> mbf_3beams_bf_addr_y_user_data_out/1
  io_delay/1 -> slice_reg/1
  slice_reg/1 -> reint1/1
  reint1/1 -> in_reg/1

== system mbf_3beams/bf_cnt_rst ==
  io_delay : xbsIndex_r4/Delay  rst=off  infoeditControl=Selection of Reset will increase slice count due to use of real FFs and instead of SRLs  en=off  latency=0  dbl_ovrd=off  reg_retiming=on  block_type=delay
  mbf_3beams_bf_cnt_rst_user_data_out : xbsIndex_r4/Gateway In  gui_display_data_type=Fixed-point  arith_type=Unsigned  n_bits=32  bin_pt=0  preci_type=Single  exp_width=8  frac_width=24  quantization="Round  (unbiased: +/- Inf)"  overflow=Saturate  period=1  dbl_ovrd=off  timing_constraint=None  locs_specified=off  LOCs={}  UseAsADC=off  ADCChannel='1'  inherit_from_input=off  hdl_port=on  block_type=gatewayin
  sim_1 : Constant  Value=0
  slice_reg : xbsIndex_r4/Slice  nbits=32  boolean_output=on  mode=Lower Bit Location + Width  bit1=0  base1=MSB of Input  bit0=0  base0=LSB of Input  dbl_ovrd=off  block_type=slice
  in_reg : Outport
  wires:
  mbf_3beams_bf_cnt_rst_user_data_out/1 -> io_delay/1
  sim_1/1 -> mbf_3beams_bf_cnt_rst_user_data_out/1
  io_delay/1 -> slice_reg/1
  slice_reg/1 -> in_reg/1

== system mbf_3beams/bf_gain ==
  io_delay : xbsIndex_r4/Delay  rst=off  infoeditControl=Selection of Reset will increase slice count due to use of real FFs and instead of SRLs  en=off  latency=0  dbl_ovrd=off  reg_retiming=on  block_type=delay
  mbf_3beams_bf_gain_user_data_out : xbsIndex_r4/Gateway In  gui_display_data_type=Fixed-point  arith_type=Unsigned  n_bits=32  bin_pt=0  preci_type=Single  exp_width=8  frac_width=24  quantization="Round  (unbiased: +/- Inf)"  overflow=Saturate  period=1  dbl_ovrd=off  timing_constraint=None  locs_specified=off  LOCs={}  UseAsADC=off  ADCChannel='1'  inherit_from_input=off  hdl_port=on  block_type=gatewayin
  reint1 : xbsIndex_r4/Reinterpret  force_arith_type=on  arith_type=Unsigned  force_bin_pt=on  bin_pt=0  block_type=reinterpret
  sim_1 : Constant  Value=0
  slice_reg : xbsIndex_r4/Slice  nbits=32  boolean_output=off  mode=Lower Bit Location + Width  bit1=0  base1=MSB of Input  bit0=0  base0=LSB of Input  dbl_ovrd=off  block_type=slice
  in_reg : Outport
  wires:
  mbf_3beams_bf_gain_user_data_out/1 -> io_delay/1
  sim_1/1 -> mbf_3beams_bf_gain_user_data_out/1
  io_delay/1 -> slice_reg/1
  slice_reg/1 -> reint1/1
  reint1/1 -> in_reg/1

== system mbf_3beams/bf_new_acc ==
  io_delay : xbsIndex_r4/Delay  rst=off  infoeditControl=Selection of Reset will increase slice count due to use of real FFs and instead of SRLs  en=off  latency=0  dbl_ovrd=off  reg_retiming=on  block_type=delay
  mbf_3beams_bf_new_acc_user_data_out : xbsIndex_r4/Gateway In  gui_display_data_type=Fixed-point  arith_type=Unsigned  n_bits=32  bin_pt=0  preci_type=Single  exp_width=8  frac_width=24  quantization="Round  (unbiased: +/- Inf)"  overflow=Saturate  period=1  dbl_ovrd=off  timing_constraint=None  locs_specified=off  LOCs={}  UseAsADC=off  ADCChannel='1'  inherit_from_input=off  hdl_port=on  block_type=gatewayin
  sim_1 : Constant  Value=0
  slice_reg : xbsIndex_r4/Slice  nbits=32  boolean_output=on  mode=Lower Bit Location + Width  bit1=0  base1=MSB of Input  bit0=0  base0=LSB of Input  dbl_ovrd=off  block_type=slice
  in_reg : Outport
  wires:
  mbf_3beams_bf_new_acc_user_data_out/1 -> io_delay/1
  sim_1/1 -> mbf_3beams_bf_new_acc_user_data_out/1
  io_delay/1 -> slice_reg/1
  slice_reg/1 -> in_reg/1

== system mbf_3beams/bf_phase_im ==
  io_delay : xbsIndex_r4/Delay  rst=off  infoeditControl=Selection of Reset will increase slice count due to use of real FFs and instead of SRLs  en=off  latency=0  dbl_ovrd=off  reg_retiming=on  block_type=delay
  mbf_3beams_bf_phase_im_user_data_out : xbsIndex_r4/Gateway In  gui_display_data_type=Fixed-point  arith_type=Unsigned  n_bits=32  bin_pt=0  preci_type=Single  exp_width=8  frac_width=24  quantization="Round  (unbiased: +/- Inf)"  overflow=Saturate  period=1  dbl_ovrd=off  timing_constraint=None  locs_specified=off  LOCs={}  UseAsADC=off  ADCChannel='1'  inherit_from_input=off  hdl_port=on  block_type=gatewayin
  reint1 : xbsIndex_r4/Reinterpret  force_arith_type=on  arith_type=Unsigned  force_bin_pt=on  bin_pt=0  block_type=reinterpret
  sim_1 : Constant  Value=0
  slice_reg : xbsIndex_r4/Slice  nbits=32  boolean_output=off  mode=Lower Bit Location + Width  bit1=0  base1=MSB of Input  bit0=0  base0=LSB of Input  dbl_ovrd=off  block_type=slice
  in_reg : Outport
  wires:
  mbf_3beams_bf_phase_im_user_data_out/1 -> io_delay/1
  sim_1/1 -> mbf_3beams_bf_phase_im_user_data_out/1
  io_delay/1 -> slice_reg/1
  slice_reg/1 -> reint1/1
  reint1/1 -> in_reg/1

== system mbf_3beams/bf_phase_re ==
  io_delay : xbsIndex_r4/Delay  rst=off  infoeditControl=Selection of Reset will increase slice count due to use of real FFs and instead of SRLs  en=off  latency=0  dbl_ovrd=off  reg_retiming=on  block_type=delay
  mbf_3beams_bf_phase_re_user_data_out : xbsIndex_r4/Gateway In  gui_display_data_type=Fixed-point  arith_type=Unsigned  n_bits=32  bin_pt=0  preci_type=Single  exp_width=8  frac_width=24  quantization="Round  (unbiased: +/- Inf)"  overflow=Saturate  period=1  dbl_ovrd=off  timing_constraint=None  locs_specified=off  LOCs={}  UseAsADC=off  ADCChannel='1'  inherit_from_input=off  hdl_port=on  block_type=gatewayin
  reint1 : xbsIndex_r4/Reinterpret  force_arith_type=on  arith_type=Unsigned  force_bin_pt=on  bin_pt=0  block_type=reinterpret
  sim_1 : Constant  Value=0
  slice_reg : xbsIndex_r4/Slice  nbits=32  boolean_output=off  mode=Lower Bit Location + Width  bit1=0  base1=MSB of Input  bit0=0  base0=LSB of Input  dbl_ovrd=off  block_type=slice
  in_reg : Outport
  wires:
  mbf_3beams_bf_phase_re_user_data_out/1 -> io_delay/1
  sim_1/1 -> mbf_3beams_bf_phase_re_user_data_out/1
  io_delay/1 -> slice_reg/1
  slice_reg/1 -> reint1/1
  reint1/1 -> in_reg/1

== system mbf_3beams/bf_phase_we ==
  io_delay : xbsIndex_r4/Delay  rst=off  infoeditControl=Selection of Reset will increase slice count due to use of real FFs and instead of SRLs  en=off  latency=0  dbl_ovrd=off  reg_retiming=on  block_type=delay
  mbf_3beams_bf_phase_we_user_data_out : xbsIndex_r4/Gateway In  gui_display_data_type=Fixed-point  arith_type=Unsigned  n_bits=32  bin_pt=0  preci_type=Single  exp_width=8  frac_width=24  quantization="Round  (unbiased: +/- Inf)"  overflow=Saturate  period=1  dbl_ovrd=off  timing_constraint=None  locs_specified=off  LOCs={}  UseAsADC=off  ADCChannel='1'  inherit_from_input=off  hdl_port=on  block_type=gatewayin
  sim_1 : Constant  Value=0
  slice_reg : xbsIndex_r4/Slice  nbits=32  boolean_output=on  mode=Lower Bit Location + Width  bit1=0  base1=MSB of Input  bit0=0  base0=LSB of Input  dbl_ovrd=off  block_type=slice
  in_reg : Outport
  wires:
  mbf_3beams_bf_phase_we_user_data_out/1 -> io_delay/1
  sim_1/1 -> mbf_3beams_bf_phase_we_user_data_out/1
  io_delay/1 -> slice_reg/1
  slice_reg/1 -> in_reg/1

== system mbf_3beams/bf_probe0 ==
  sync : Inport
  s0 : Inport  Port=2
  s1 : Inport  Port=3
  s2 : Inport  Port=4
  gain : Inport  Port=5
  we : Inport  Port=6
  addr : Inport  Port=7
  new_acc : Inport  Port=8
  pipeline1 : Subsystem  AncestorBlock=casper_library_delays/pipeline  mask(pipeline){latency=1}
      mask help:
      | An explicitly laid-out delay line to avoid mapping to SRL16s
      | for use in pipelining to help achieve timing closure.
      mask init:
      | pipeline_init(gcb,'latency',latency);
      settings: AttributesFormatString=Latency = %<latency>
      mask Display:
      | port_label('input', 1, 'd');
      | port_label('output', 1, 'q');
      | disp(['Z^-', num2str(latency)]);
  pipeline2 : Subsystem  AncestorBlock=casper_library_delays/pipeline  mask(pipeline){latency=1}
      mask help:
      | An explicitly laid-out delay line to avoid mapping to SRL16s
      | for use in pipelining to help achieve timing closure.
      mask init:
      | pipeline_init(gcb,'latency',latency);
      settings: AttributesFormatString=Latency = %<latency>
      mask Display:
      | port_label('input', 1, 'd');
      | port_label('output', 1, 'q');
      | disp(['Z^-', num2str(latency)]);
  pipeline3 : Subsystem  AncestorBlock=casper_library_delays/pipeline  mask(pipeline){latency=1}
      mask help:
      | An explicitly laid-out delay line to avoid mapping to SRL16s
      | for use in pipelining to help achieve timing closure.
      mask init:
      | pipeline_init(gcb,'latency',latency);
      settings: AttributesFormatString=Latency = %<latency>
      mask Display:
      | port_label('input', 1, 'd');
      | port_label('output', 1, 'q');
      | disp(['Z^-', num2str(latency)]);
  pipeline41 : Subsystem  AncestorBlock=casper_library_delays/pipeline  mask(pipeline){latency=1}
      mask help:
      | An explicitly laid-out delay line to avoid mapping to SRL16s
      | for use in pipelining to help achieve timing closure.
      mask init:
      | pipeline_init(gcb,'latency',latency);
      settings: AttributesFormatString=Latency = %<latency>
      mask Display:
      | port_label('input', 1, 'd');
      | port_label('output', 1, 'q');
      | disp(['Z^-', num2str(latency)]);
  pipeline42 : Subsystem  AncestorBlock=casper_library_delays/pipeline  mask(pipeline){latency=1}
      mask help:
      | An explicitly laid-out delay line to avoid mapping to SRL16s
      | for use in pipelining to help achieve timing closure.
      mask init:
      | pipeline_init(gcb,'latency',latency);
      settings: AttributesFormatString=Latency = %<latency>
      mask Display:
      | port_label('input', 1, 'd');
      | port_label('output', 1, 'q');
      | disp(['Z^-', num2str(latency)]);
  pipeline43 : Subsystem  AncestorBlock=casper_library_delays/pipeline  mask(pipeline){latency=1}
      mask help:
      | An explicitly laid-out delay line to avoid mapping to SRL16s
      | for use in pipelining to help achieve timing closure.
      mask init:
      | pipeline_init(gcb,'latency',latency);
      settings: AttributesFormatString=Latency = %<latency>
      mask Display:
      | port_label('input', 1, 'd');
      | port_label('output', 1, 'q');
      | disp(['Z^-', num2str(latency)]);
  pipeline58 : Subsystem  AncestorBlock=casper_library_delays/pipeline  mask(pipeline){latency=1}
      mask help:
      | An explicitly laid-out delay line to avoid mapping to SRL16s
      | for use in pipelining to help achieve timing closure.
      mask init:
      | pipeline_init(gcb,'latency',latency);
      settings: AttributesFormatString=Latency = %<latency>
      mask Display:
      | port_label('input', 1, 'd');
      | port_label('output', 1, 'q');
      | disp(['Z^-', num2str(latency)]);
  pipeline61 : Subsystem  AncestorBlock=casper_library_delays/pipeline  mask(pipeline){latency=1}
      mask help:
      | An explicitly laid-out delay line to avoid mapping to SRL16s
      | for use in pipelining to help achieve timing closure.
      mask init:
      | pipeline_init(gcb,'latency',latency);
      settings: AttributesFormatString=Latency = %<latency>
      mask Display:
      | port_label('input', 1, 'd');
      | port_label('output', 1, 'q');
      | disp(['Z^-', num2str(latency)]);
  pipeline62 : Subsystem  AncestorBlock=casper_library_delays/pipeline  mask(pipeline){latency=1}
      mask help:
      | An explicitly laid-out delay line to avoid mapping to SRL16s
      | for use in pipelining to help achieve timing closure.
      mask init:
      | pipeline_init(gcb,'latency',latency);
      settings: AttributesFormatString=Latency = %<latency>
      mask Display:
      | port_label('input', 1, 'd');
      | port_label('output', 1, 'q');
      | disp(['Z^-', num2str(latency)]);
  pow_quant : Subsystem
  xpow : Subsystem
  sync_out : Outport
  bf_valid : Outport  Port=2
  wires:
  we/1 -> xpow/1(we)
  addr/1 -> xpow/2(addr)
  new_acc/1 -> xpow/3(new_acc)
  sync/1 -> pipeline62/1(d)
  s0/1 -> pipeline58/1(d)
  s1/1 -> pipeline61/1(d)
  gain/1 -> pipeline1/1(d)
  pow_quant/1(sync_out) -> pipeline43/1(d)
  pow_quant/2(out0) -> pipeline42/1(d)
  pow_quant/3(out1) -> pipeline41/1(d)
  pipeline43/1(q) -> sync_out/1
  pipeline42/1(q) -> xpow/4(din1)
  pipeline41/1(q) -> xpow/5(din2)
  pipeline62/1(q) -> pow_quant/1(sync)
  pipeline61/1(q) -> pow_quant/3(in1)
  pipeline58/1(q) -> pow_quant/2(in0)
  xpow/1(valid) -> bf_valid/1
  pipeline1/1(q) -> pow_quant/5(gain)
  pipeline2/1(q) -> pow_quant/4(in2)
  s2/1 -> pipeline2/1(d)
  pow_quant/4(out2) -> pipeline3/1(d)
  pipeline3/1(q) -> xpow/6(din3)

== system mbf_3beams/cal_acc_len ==
  io_delay : xbsIndex_r4/Delay  rst=off  infoeditControl=Selection of Reset will increase slice count due to use of real FFs and instead of SRLs  en=off  latency=0  dbl_ovrd=off  reg_retiming=on  block_type=delay
  mbf_3beams_cal_acc_len_user_data_out : xbsIndex_r4/Gateway In  gui_display_data_type=Fixed-point  arith_type=Unsigned  n_bits=32  bin_pt=0  preci_type=Single  exp_width=8  frac_width=24  quantization="Round  (unbiased: +/- Inf)"  overflow=Saturate  period=1  dbl_ovrd=off  timing_constraint=None  locs_specified=off  LOCs={}  UseAsADC=off  ADCChannel='1'  inherit_from_input=off  hdl_port=on  block_type=gatewayin
  reint1 : xbsIndex_r4/Reinterpret  force_arith_type=on  arith_type=Unsigned  force_bin_pt=on  bin_pt=0  block_type=reinterpret
  sim_1 : Constant  Value=0
  slice_reg : xbsIndex_r4/Slice  nbits=32  boolean_output=off  mode=Lower Bit Location + Width  bit1=0  base1=MSB of Input  bit0=0  base0=LSB of Input  dbl_ovrd=off  block_type=slice
  in_reg : Outport
  wires:
  mbf_3beams_cal_acc_len_user_data_out/1 -> io_delay/1
  sim_1/1 -> mbf_3beams_cal_acc_len_user_data_out/1
  io_delay/1 -> slice_reg/1
  slice_reg/1 -> reint1/1
  reint1/1 -> in_reg/1

== system mbf_3beams/cal_cnt_rst ==
  io_delay : xbsIndex_r4/Delay  rst=off  infoeditControl=Selection of Reset will increase slice count due to use of real FFs and instead of SRLs  en=off  latency=0  dbl_ovrd=off  reg_retiming=on  block_type=delay
  mbf_3beams_cal_cnt_rst_user_data_out : xbsIndex_r4/Gateway In  gui_display_data_type=Fixed-point  arith_type=Unsigned  n_bits=32  bin_pt=0  preci_type=Single  exp_width=8  frac_width=24  quantization="Round  (unbiased: +/- Inf)"  overflow=Saturate  period=1  dbl_ovrd=off  timing_constraint=None  locs_specified=off  LOCs={}  UseAsADC=off  ADCChannel='1'  inherit_from_input=off  hdl_port=on  block_type=gatewayin
  sim_1 : Constant  Value=0
  slice_reg : xbsIndex_r4/Slice  nbits=32  boolean_output=on  mode=Lower Bit Location + Width  bit1=0  base1=MSB of Input  bit0=0  base0=LSB of Input  dbl_ovrd=off  block_type=slice
  in_reg : Outport
  wires:
  mbf_3beams_cal_cnt_rst_user_data_out/1 -> io_delay/1
  sim_1/1 -> mbf_3beams_cal_cnt_rst_user_data_out/1
  io_delay/1 -> slice_reg/1
  slice_reg/1 -> in_reg/1

== system mbf_3beams/cal_gain ==
  io_delay : xbsIndex_r4/Delay  rst=off  infoeditControl=Selection of Reset will increase slice count due to use of real FFs and instead of SRLs  en=off  latency=0  dbl_ovrd=off  reg_retiming=on  block_type=delay
  mbf_3beams_cal_gain_user_data_out : xbsIndex_r4/Gateway In  gui_display_data_type=Fixed-point  arith_type=Unsigned  n_bits=32  bin_pt=0  preci_type=Single  exp_width=8  frac_width=24  quantization="Round  (unbiased: +/- Inf)"  overflow=Saturate  period=1  dbl_ovrd=off  timing_constraint=None  locs_specified=off  LOCs={}  UseAsADC=off  ADCChannel='1'  inherit_from_input=off  hdl_port=on  block_type=gatewayin
  reint1 : xbsIndex_r4/Reinterpret  force_arith_type=on  arith_type=Unsigned  force_bin_pt=on  bin_pt=0  block_type=reinterpret
  sim_1 : Constant  Value=0
  slice_reg : xbsIndex_r4/Slice  nbits=32  boolean_output=off  mode=Lower Bit Location + Width  bit1=0  base1=MSB of Input  bit0=0  base0=LSB of Input  dbl_ovrd=off  block_type=slice
  in_reg : Outport
  wires:
  mbf_3beams_cal_gain_user_data_out/1 -> io_delay/1
  sim_1/1 -> mbf_3beams_cal_gain_user_data_out/1
  io_delay/1 -> slice_reg/1
  slice_reg/1 -> reint1/1
  reint1/1 -> in_reg/1
